simulink model "GLOSA_cs"  (Simulink 7.6)
settings: Solver=ode5  SolverMode=SingleTasking  StartTime=0.0  StopTime=40  FixedStep=1/100  RelTol=1e-3
PreLoadFcn:
  | check_NUTS_defined;
  | 
  | assignin('base',['model_closed_' bdroot],false);
InitFcn:
  | PreScanExperimentInitFcn(bdroot)
StopFcn:
  | PreScanExperimentStopFcn(bdroot)
undefined symbols (not defined in the file or in its folder): acceleration, actorID, actorType, DiscIntNeverNeededParam, GPS_alt, GPS_lat, GPS_long, intmax, lightBarInUse, messageID, publicVehicleType, rotZ, sireneInUse, trafficLightPriority, turnAdvice, velocity, x_ini_VRML, y_ini_VRML, yawRate, z_ini_VRML
libraries: simulink x337, simulink_extras x42, PreScanSimulinkLibrary x38, PreScanUsefulBlocks x14, simulink_need_slupdate x6
(part 37 of 38)

== stateflow chart BMW_Z3_1/GLOSA/GLOSA ==
  state GLOSA [parallel]
    settings: executionOrder=1
    state dvVelocity
      (default) -> (junction 445) : /* Velocity */
      (junction 445) -> (junction 447) [order 1] : [ (vForward == 1)]
      (junction 447) -> (junction 457) : {dvVelocity = CAM_Velocity[i];}
          | /* If car is at the front desired velocity if cars are set to its value */
      (junction 445) -> (junction 391) [order 2] : {dvVelocity = desiredVelocity;}
      (junction 457) -> (junction 391)
      (junction 391) -> (junction 458)
    state AngleandDistance
      state vDistance [parallel]
        settings: executionOrder=2
        (junction 439) -> (junction 438) : {Distance = 6371000*c;}
            | /*Calculate distance to the object */
        (default) -> (junction 440) : {a = (sin((CAM_GPSLatitude[i]*d2r-selfGPSLatitude*d2r)/2 ))^2+ cos(selfGPSLatitude*d2r)*cos(CAM_GPSLatitude[i]*d2r)*(sin((CAM_GPSLongitude[i]*d2r-selfGPSLongitude*d2r)/2))^2;}
        (junction 440) -> (junction 439) : {c=2*atan2(sqrt(a), sqrt(1-a));}
      state vAngle [parallel]
        settings: executionOrder=1
        (default) -> (junction 441) : {Angle = (atan2(CAM_GPSLatitude[i]-selfGPSLatitude, CAM_GPSLongitude[i] - selfGPSLongitude))/d2r;}
            | /* Calculate angle to the object */
    state diVelocity
      (default) -> (junction 412) : /* Velocity */
      (junction 393) -> (junction 446)
      (junction 412) -> (junction 411) [order 1] : [ (iForward == 1)]
      (junction 412) -> (junction 393) [order 2] : {diVelocity = desiredVelocity;}
      (junction 411) -> (junction 444) : {diVelocity = (CAM_message[i] - 1000) /3.6;}
          | /* If sign is foreward, set desired infrastructure velocity to its limit */
      (junction 444) -> (junction 393)
    state vForward
      (junction 397) -> (junction 403) [order 1] : [((CAM_YawRate[i] > 2 || CAM_YawRate[i] <-2)  && (selfYawRate > 2 || selfYawRate < -2))]
      (junction 403) -> (junction 427) : {R1 = CAM_Velocity[i]/(CAM_YawRate[i]*d2r);
          | R2 = selfVelocity/(selfYawRate*d2r);
          | alfa = CAM_Heading[i]-selfHeading;
          | L = 2*d2r*180*R2*(alfa/360);}
      (junction 464) -> (junction 449)
      (junction 448) -> (junction 464) : {bend = 1;}
          | /* Cars are on the same bent if both have non-zero yaw rate and estimated agle at detected distance of the host car is similar to the angle of detected vehicle */
      (junction 427) -> (junction 449) [order 2] : {bend = 2;}
      (junction 387) -> (junction 390)
      (junction 401) -> (junction 388) [order 2]
      (junction 449) -> (junction 402)
      (junction 402) -> (junction 401)
      (junction 390) -> (junction 388) [order 2]
      (default) -> (junction 397)
      (junction 394) -> (junction 395) [order 1] : [abs((Angle-selfHeading)%%360)<45 || abs((Angle-selfHeading)%%360)>315 || bend == 1]
      (junction 443) -> (junction 435) : {vForward = 0;}
      (junction 395) -> (junction 396) : {vForward = 1;}
          | /* Car is at the front if it will be reached in 2s and angles of cars are similar or they are on the bend */
      (junction 401) -> (junction 387) [order 1] : [(abs(CAM_Heading[i]-selfHeading)<45 || abs(CAM_Heading[i]-selfHeading)>315) || bend == 1 ]
      (junction 394) -> (junction 443) [order 2]
      (junction 390) -> (junction 392) [order 1] : [Distance < 100 && (selfVelocity - CAM_Velocity[i]) > 0 &&((Distance / (selfVelocity - CAM_Velocity[i])) < 2) && (abs(selfGPSAltitude - CAM_GPSAltitude[i]) < 10)]
      (junction 397) -> (junction 402) [order 2] : {bend = 0;}
      (junction 392) -> (junction 394)
      (junction 388) -> (junction 443)
      (junction 427) -> (junction 448) [order 1] : [((abs(L/selfVelocity*selfYawRate + selfHeading - CAM_Heading[i]) < 45) || (abs(L/selfVelocity*selfYawRate + selfHeading - CAM_Heading[i]) > 315)) ]
    state Velocity
      (junction 417) -> (junction 415) [order 2]
      (junction 417) -> (junction 416) [order 1] : [(dvVelocity<diVelocity) && (dvVelocity < desiredVelocity) && (vForward == 1)]
      (junction 419) -> (junction 417)
      (junction 418) -> (junction 419)
      (junction 433) -> (junction 418) : {Velocity = diVelocity;}
          | /* When Velocity from the sign is smaller then actual velocity of the car, Desired host velocity is set to the sign limit */
      (junction 434) -> (junction 419) [order 2] : {Velocity = desiredVelocity;}
      (junction 434) -> (junction 433) [order 1] : [(diVelocity<desiredVelocity)]
      (default) -> (junction 434) : /* Velocity */
      (junction 416) -> (junction 414) : {Velocity = dvVelocity;}
          | /* When detected car hes smaller velocity then host car and sign limits, desired velocity of host car is set to velocity of detected car */
      (junction 415) -> (junction 413) : {Velocity = min(dvVelocity, diVelocity, dlVelocity, desiredVelocity);}
      (junction 414) -> (junction 415)
    state lForward
      note: IF condition1 THEN action1
          | ELSE action2
          | ENDIF
      (junction 454) -> (junction 399) : {lForward = 0;}
      (junction 470) -> (junction 400) : {lForward = 1;}
          | /* Light recognized as foreward one, when it is less then 500m away and Angle to it less then 45 deg.*/
      (junction 452) -> (junction 470) [order 1] : [abs((Angle-selfHeading) %% 360)<45 || abs((Angle-selfHeading)%% 360)>315]
      (junction 452) -> (junction 454) [order 2]
      (junction 467) -> (junction 450) [order 2]
      (junction 451) -> (junction 450) [order 2]
      (junction 456) -> (junction 452)
      (junction 466) -> (junction 451)
      (junction 451) -> (junction 456) [order 1] : [Distance < 500 && (abs(selfGPSAltitude - CAM_GPSAltitude[i]) < 10)]
      (junction 450) -> (junction 454)
      (junction 467) -> (junction 466) [order 1] : [abs(CAM_Heading[i]-selfHeading)<45 || abs(CAM_Heading[i]-selfHeading)>315]
      (default) -> (junction 467)
    state iForward
      note: IF condition1 THEN action1
          | ELSE action2
          | ENDIF
      (junction 389) -> (junction 436) [order 1] : [Distance < 20 && (abs(selfGPSAltitude - CAM_GPSAltitude[i]) < 10)]
      (junction 398) -> (junction 424) [order 1] : [abs((Angle-selfHeading)%%360)<45 || abs((Angle-selfHeading)%%360)>315]
      (junction 389) -> (junction 423) [order 2]
      (junction 424) -> (junction 425) : {iForward = 1;}
          | /* Sign recognized as foreward one, when it is less then 20m away and Angle to it less then 45 deg.*/
      (junction 423) -> (junction 437)
      (junction 436) -> (junction 398)
      (junction 437) -> (junction 426) : {iForward = 0;}
      (junction 398) -> (junction 437) [order 2]
      (junction 442) -> (junction 432) [order 1] : [abs(CAM_Heading[i]-selfHeading)<45 || abs(CAM_Heading[i]-selfHeading)>315]
      (default) -> (junction 442)
      (junction 432) -> (junction 389)
      (junction 442) -> (junction 423) [order 2]
    state dlVelocity
      (junction 430) -> (junction 428) : {dlVelocity = max((Distance-7)/(CAM_warning[i]+20), 0);}
          | /*dlVelocity = Distance/CAM_warning[i];}*/
      (junction 461) -> (junction 431) [order 2]
      (junction 465) -> (junction 468)
      (junction 469) -> (junction 465)
      (junction 459) -> (junction 465) [order 2] : {dlVelocity=desiredVelocity;}
      (junction 455) -> (junction 453)
      (junction 463) -> (junction 461) [order 2]
      (junction 428) -> (junction 469)
      (junction 431) -> (junction 430) [order 2]
      (junction 431) -> (junction 429) [order 1] : [TTL<CAM_warning[i]]
      (junction 463) -> (junction 462) [order 1] : [CAM_trafficLightPriority[i] == 3]
      (junction 429) -> (junction 453) : {dlVelocity= desiredVelocity;}
      (junction 405) -> (junction 455)
      (junction 453) -> (junction 428)
      (junction 462) -> (junction 405) : {dlVelocity=desiredVelocity;}
      (junction 404) -> (junction 455) : {dlVelocity = max((Distance-2)/(CAM_warning[i]+0.5), 0);}
      (junction 461) -> (junction 404) [order 1] : [CAM_trafficLightPriority[i] == 1]
      (default) -> (junction 459) : /* Velocity */
      (junction 459) -> (junction 460) [order 1] : [ lForward == 1]
      (junction 460) -> (junction 463) : {TTL = Distance/selfVelocity;
          | Light = CAM_trafficLightPriority[i];
          | Time = CAM_warning[i];}
    lForward -> dlVelocity
    (junction 407) -> AngleandDistance
    (default) -> (junction 406) : {i =0;
        | vForward = 0;
        | iForward = 0;}
    (junction 406) -> (junction 407) [order 1] : [(vForward ==0 || iForward == 0) && i < CAM_Size]
        |  /*till sign and vehicle aren't detected*/
    (junction 408) -> iForward [order 2] : [iForward == 0 && (CAM_vehicleType[i] == 14 || CAM_vehicleType[i] == 15) && CAM_message[i] > 1000]
        | /* If no traffic sign are detected and Velocity of the sign isn't 0 */
    (junction 410) -> (junction 406) : {i = 0;
        | vForward = 0;
        | iForward = 0;
        | lForward = 0;}
    (junction 420) -> (junction 406) : {i++;}
    (junction 408) -> (junction 409) [order 3]
    (junction 406) -> Velocity [order 2]
    (junction 409) -> vForward [order 1] : [vForward == 0 && CAM_vehicleType[i] != 0]
        | /* If there was no nearer car at the front and Vehicle Type ID isn't 0 */
    (junction 409) -> (junction 420) [order 2]
    iForward -> diVelocity
    Velocity -> (junction 410)
    vForward -> dvVelocity
    dvVelocity -> (junction 422)
    diVelocity -> (junction 422)
    (junction 421) -> (junction 420)
    (junction 422) -> (junction 421)
    AngleandDistance -> (junction 408)
    (junction 408) -> lForward [order 1] : [lForward == 0 && CAM_trafficLightPriority[i] !=0]
    dlVelocity -> (junction 422)
  note: GLOSA Algorithms Based on CAM signal changed to continous
  data: R1 local double; R2 local double; a local double; alfa local double; bend local double; c local double; diVelocity local double =100; dvVelocity local double =100; i local double; iForward local double; L local double; vForward local double; dlVelocity local double =100; selfHeading input double; selfGPSLongitude input double; selfGPSLatitude input double; selfGPSAltitude input double; selfYawRate input double; selfVelocity input double; CAM_warning input double; CAM_message input double; CAM_vehicleType input double; Velocity output double; CAM_Velocity input double; CAM_YawRate input double; CAM_trafficLightPriority input double; CAM_GPSLatitude input double; CAM_GPSLongitude input double; CAM_GPSAltitude input double; CAM_Heading input double; CAM_Size input double; d2r constant double =pi()/180; desiredVelocity input double; TTL local double; lForward output double; Angle local double; Distance local double; Time output double; Light output double

== matlab function BMW_Z3_1/GLOSA/vehicle CAM generator/For Iterator Subsystem/Continuous signal ==
  data: CAM input double; CAM_o output double; CAM_old input double; i input double; NoMM input double; tmp_in input double; tmp_out output double
function [CAM_o, tmp_out] = con(CAM, CAM_old, i, NoMM, tmp_in)
%linearization and number of missed frames control
CAM_o = zeros(1, 16);
tmp_out = tmp_in(i);
if (CAM(i, 3) ~= 0)
    if (mod(tmp_in(i), NoMM+1) == 0)
        CAM_o = CAM (i, :);
    end
    tmp_out = tmp_in(i)+1;
else
    CAM_o = CAM_old(i, :);
end

== matlab function BMW_Z3_1/GLOSA/vehicle CAM generator/Seperate signals ==
  data: CAM_warning output double; CAM input double; CAM_messageID output double; CAM_vehicleID output double; CAM_vehicleType output double; CAM_publicVehicleType output double; CAM_lightBarInUse output double; CAM_sireneInUse output double; CAM_velocity output double; CAM_acceleration output double; CAM_yawRate output double; CAM_turnAdvice output double; CAM_trafficLightPriority output double; CAM_GPSlat output double; CAM_GPSlong output double; CAM_GPSalt output double; CAM_rotZ output double
function [CAM_warning, CAM_messageID, CAM_vehicleID, CAM_vehicleType, CAM_publicVehicleType, CAM_lightBarInUse, CAM_sireneInUse, CAM_velocity, CAM_acceleration, CAM_yawRate, CAM_turnAdvice, CAM_trafficLightPriority, CAM_GPSlat, CAM_GPSlong, CAM_GPSalt, CAM_rotZ] = fcn(CAM)
%Seperate signals

CAM_warning = CAM(:, 1);
CAM_messageID = CAM(:, 2); 
CAM_vehicleID = CAM(:, 3);
CAM_vehicleType = CAM(:, 4);
CAM_publicVehicleType = CAM(:, 5);
CAM_lightBarInUse = CAM(:, 6);
CAM_sireneInUse = CAM(:, 7);
CAM_velocity = CAM(:, 8);
CAM_acceleration = CAM(:, 9);
CAM_yawRate = CAM(:, 10);
CAM_turnAdvice = CAM(:, 11);
CAM_trafficLightPriority = CAM(:, 12);
CAM_GPSlat = CAM(:, 13);
CAM_GPSlong = CAM(:, 14);
CAM_GPSalt = CAM(:, 15);
CAM_rotZ = CAM(:, 16);

== matlab function BMW_Z3_1/GLOSA/vehicle CAM generator/Signal Matrix create ==
  data: CAM_warning input double; CAM output double; CAM_messageID input double; CAM_vehicleID input double; CAM_vehicleType input double; CAM_publicVehicleType input double; CAM_lightBarInUse input double; CAM_sireneInUse input double; CAM_velocity input double; CAM_acceleration input double; CAM_yawRate input double; CAM_turnAdvice input double; CAM_trafficLightPriority input double; CAM_GPSlat input double; CAM_GPSlong input double; CAM_GPSalt input double; CAM_rotZ input double
function CAM = fcn(CAM_warning, CAM_messageID, CAM_vehicleID, CAM_vehicleType, CAM_publicVehicleType, CAM_lightBarInUse, CAM_sireneInUse, CAM_velocity, CAM_acceleration, CAM_yawRate, CAM_turnAdvice, CAM_trafficLightPriority, CAM_GPSlat, CAM_GPSlong, CAM_GPSalt, CAM_rotZ)
%Signal Matrix creation

CAM = [CAM_warning, CAM_messageID, CAM_vehicleID, CAM_vehicleType, CAM_publicVehicleType, CAM_lightBarInUse, CAM_sireneInUse, CAM_velocity, CAM_acceleration, CAM_yawRate, CAM_turnAdvice, CAM_trafficLightPriority, CAM_GPSlat, CAM_GPSlong, CAM_GPSalt, CAM_rotZ];

== matlab function Toyota_Yaris_1/GLOSA/Embedded MATLAB Function ==
  data: u input double; y output double
function y = fcn(u)
%#eml

y = mod(u, 360);

== stateflow chart Toyota_Yaris_1/GLOSA/GLOSA ==
  state GLOSA [parallel]
    settings: executionOrder=1
    state dvVelocity
      (default) -> (junction 760) : /* Velocity */
      (junction 760) -> (junction 762) [order 1] : [ (vForward == 1)]
      (junction 762) -> (junction 772) : {dvVelocity = CAM_Velocity[i];}
          | /* If car is at the front desired velocity if cars are set to its value */
      (junction 760) -> (junction 706) [order 2] : {dvVelocity = desiredVelocity;}
      (junction 772) -> (junction 706)
      (junction 706) -> (junction 773)
    state AngleandDistance
      state vDistance [parallel]
        settings: executionOrder=2
        (junction 754) -> (junction 753) : {Distance = 6371000*c;}
            | /*Calculate distance to the object */
        (default) -> (junction 755) : {a = (sin((CAM_GPSLatitude[i]*d2r-selfGPSLatitude*d2r)/2 ))^2+ cos(selfGPSLatitude*d2r)*cos(CAM_GPSLatitude[i]*d2r)*(sin((CAM_GPSLongitude[i]*d2r-selfGPSLongitude*d2r)/2))^2;}
        (junction 755) -> (junction 754) : {c=2*atan2(sqrt(a), sqrt(1-a));}
      state vAngle [parallel]
        settings: executionOrder=1
        (default) -> (junction 756) : {Angle = (atan2(CAM_GPSLatitude[i]-selfGPSLatitude, CAM_GPSLongitude[i] - selfGPSLongitude))/d2r;}
            | /* Calculate angle to the object */
    state diVelocity
      (default) -> (junction 727) : /* Velocity */
      (junction 708) -> (junction 761)
      (junction 727) -> (junction 726) [order 1] : [ (iForward == 1)]
      (junction 727) -> (junction 708) [order 2] : {diVelocity = desiredVelocity;}
      (junction 726) -> (junction 759) : {diVelocity = (CAM_message[i] - 1000) /3.6;}
          | /* If sign is foreward, set desired infrastructure velocity to its limit */
      (junction 759) -> (junction 708)
    state vForward
      (junction 712) -> (junction 718) [order 1] : [((CAM_YawRate[i] > 2 || CAM_YawRate[i] <-2)  && (selfYawRate > 2 || selfYawRate < -2))]
      (junction 718) -> (junction 742) : {R1 = CAM_Velocity[i]/(CAM_YawRate[i]*d2r);
          | R2 = selfVelocity/(selfYawRate*d2r);
          | alfa = CAM_Heading[i]-selfHeading;
          | L = 2*d2r*180*R2*(alfa/360);}
      (junction 779) -> (junction 764)
      (junction 763) -> (junction 779) : {bend = 1;}
          | /* Cars are on the same bent if both have non-zero yaw rate and estimated agle at detected distance of the host car is similar to the angle of detected vehicle */
      (junction 742) -> (junction 764) [order 2] : {bend = 2;}
      (junction 702) -> (junction 705)
      (junction 716) -> (junction 703) [order 2]
      (junction 764) -> (junction 717)
      (junction 717) -> (junction 716)
      (junction 705) -> (junction 703) [order 2]
      (default) -> (junction 712)
      (junction 709) -> (junction 710) [order 1] : [abs((Angle-selfHeading)%%360)<45 || abs((Angle-selfHeading)%%360)>315 || bend == 1]
      (junction 758) -> (junction 750) : {vForward = 0;}
      (junction 710) -> (junction 711) : {vForward = 1;}
          | /* Car is at the front if it will be reached in 2s and angles of cars are similar or they are on the bend */
      (junction 716) -> (junction 702) [order 1] : [(abs(CAM_Heading[i]-selfHeading)<45 || abs(CAM_Heading[i]-selfHeading)>315) || bend == 1 ]
      (junction 709) -> (junction 758) [order 2]
      (junction 705) -> (junction 707) [order 1] : [Distance < 100 && (selfVelocity - CAM_Velocity[i]) > 0 &&((Distance / (selfVelocity - CAM_Velocity[i])) < 2) && (abs(selfGPSAltitude - CAM_GPSAltitude[i]) < 10)]
      (junction 712) -> (junction 717) [order 2] : {bend = 0;}
      (junction 707) -> (junction 709)
      (junction 703) -> (junction 758)
      (junction 742) -> (junction 763) [order 1] : [((abs(L/selfVelocity*selfYawRate + selfHeading - CAM_Heading[i]) < 45) || (abs(L/selfVelocity*selfYawRate + selfHeading - CAM_Heading[i]) > 315)) ]
    state Velocity
      (junction 732) -> (junction 730) [order 2]
      (junction 732) -> (junction 731) [order 1] : [(dvVelocity<diVelocity) && (dvVelocity < desiredVelocity) && (vForward == 1)]
      (junction 734) -> (junction 732)
      (junction 733) -> (junction 734)
      (junction 748) -> (junction 733) : {Velocity = diVelocity;}
          | /* When Velocity from the sign is smaller then actual velocity of the car, Desired host velocity is set to the sign limit */
      (junction 749) -> (junction 734) [order 2] : {Velocity = desiredVelocity;}
      (junction 749) -> (junction 748) [order 1] : [(diVelocity<desiredVelocity)]
      (default) -> (junction 749) : /* Velocity */
      (junction 731) -> (junction 729) : {Velocity = dvVelocity;}
          | /* When detected car hes smaller velocity then host car and sign limits, desired velocity of host car is set to velocity of detected car */
      (junction 730) -> (junction 728) : {Velocity = min(dvVelocity, diVelocity, dlVelocity, desiredVelocity);}
      (junction 729) -> (junction 730)
    state lForward
      note: IF condition1 THEN action1
          | ELSE action2
          | ENDIF
      (junction 769) -> (junction 714) : {lForward = 0;}
      (junction 785) -> (junction 715) : {lForward = 1;}
          | /* Light recognized as foreward one, when it is less then 500m away and Angle to it less then 45 deg.*/
      (junction 767) -> (junction 785) [order 1] : [abs((Angle-selfHeading) %% 360)<45 || abs((Angle-selfHeading)%% 360)>315]
      (junction 767) -> (junction 769) [order 2]
      (junction 782) -> (junction 765) [order 2]
      (junction 766) -> (junction 765) [order 2]
      (junction 771) -> (junction 767)
      (junction 781) -> (junction 766)
      (junction 766) -> (junction 771) [order 1] : [Distance < 500 && (abs(selfGPSAltitude - CAM_GPSAltitude[i]) < 10)]
      (junction 765) -> (junction 769)
      (junction 782) -> (junction 781) [order 1] : [abs(CAM_Heading[i]-selfHeading)<45 || abs(CAM_Heading[i]-selfHeading)>315]
      (default) -> (junction 782)
    state iForward
      note: IF condition1 THEN action1
          | ELSE action2
          | ENDIF
      (junction 704) -> (junction 751) [order 1] : [Distance < 20 && (abs(selfGPSAltitude - CAM_GPSAltitude[i]) < 10)]
      (junction 713) -> (junction 739) [order 1] : [abs((Angle-selfHeading)%%360)<45 || abs((Angle-selfHeading)%%360)>315]
      (junction 704) -> (junction 738) [order 2]
      (junction 739) -> (junction 740) : {iForward = 1;}
          | /* Sign recognized as foreward one, when it is less then 20m away and Angle to it less then 45 deg.*/
      (junction 738) -> (junction 752)
      (junction 751) -> (junction 713)
      (junction 752) -> (junction 741) : {iForward = 0;}
      (junction 713) -> (junction 752) [order 2]
      (junction 757) -> (junction 747) [order 1] : [abs(CAM_Heading[i]-selfHeading)<45 || abs(CAM_Heading[i]-selfHeading)>315]
      (default) -> (junction 757)
      (junction 747) -> (junction 704)
      (junction 757) -> (junction 738) [order 2]
    state dlVelocity
      (junction 745) -> (junction 743) : {dlVelocity = max((Distance-7)/(CAM_warning[i]+20), 0);}
          | /*dlVelocity = Distance/CAM_warning[i];}*/
      (junction 776) -> (junction 746) [order 2]
      (junction 780) -> (junction 783)
      (junction 784) -> (junction 780)
      (junction 774) -> (junction 780) [order 2] : {dlVelocity=desiredVelocity;}
      (junction 770) -> (junction 768)
      (junction 778) -> (junction 776) [order 2]
      (junction 743) -> (junction 784)
      (junction 746) -> (junction 745) [order 2]
      (junction 746) -> (junction 744) [order 1] : [TTL<CAM_warning[i]]
      (junction 778) -> (junction 777) [order 1] : [CAM_trafficLightPriority[i] == 3]
      (junction 744) -> (junction 768) : {dlVelocity= desiredVelocity;}
      (junction 720) -> (junction 770)
      (junction 768) -> (junction 743)
      (junction 777) -> (junction 720) : {dlVelocity=desiredVelocity;}
      (junction 719) -> (junction 770) : {dlVelocity = max((Distance-2)/(CAM_warning[i]+0.5), 0);}
      (junction 776) -> (junction 719) [order 1] : [CAM_trafficLightPriority[i] == 1]
      (default) -> (junction 774) : /* Velocity */
      (junction 774) -> (junction 775) [order 1] : [ lForward == 1]
      (junction 775) -> (junction 778) : {TTL = Distance/selfVelocity;
          | Light = CAM_trafficLightPriority[i];
          | Time = CAM_warning[i];}
    lForward -> dlVelocity
    (junction 722) -> AngleandDistance
    (default) -> (junction 721) : {i =0;
        | vForward = 0;
        | iForward = 0;}
    (junction 721) -> (junction 722) [order 1] : [(vForward ==0 || iForward == 0) && i < CAM_Size]
        |  /*till sign and vehicle aren't detected*/
    (junction 723) -> iForward [order 2] : [iForward == 0 && (CAM_vehicleType[i] == 14 || CAM_vehicleType[i] == 15) && CAM_message[i] > 1000]
        | /* If no traffic sign are detected and Velocity of the sign isn't 0 */
    (junction 725) -> (junction 721) : {i = 0;
        | vForward = 0;
        | iForward = 0;
        | lForward = 0;}
    (junction 735) -> (junction 721) : {i++;}
    (junction 723) -> (junction 724) [order 3]
    (junction 721) -> Velocity [order 2]
    (junction 724) -> vForward [order 1] : [vForward == 0 && CAM_vehicleType[i] != 0]
        | /* If there was no nearer car at the front and Vehicle Type ID isn't 0 */
    (junction 724) -> (junction 735) [order 2]
    iForward -> diVelocity
    Velocity -> (junction 725)
    vForward -> dvVelocity
    dvVelocity -> (junction 737)
    diVelocity -> (junction 737)
    (junction 736) -> (junction 735)
    (junction 737) -> (junction 736)
    AngleandDistance -> (junction 723)
    (junction 723) -> lForward [order 1] : [lForward == 0 && CAM_trafficLightPriority[i] !=0]
    dlVelocity -> (junction 737)
  note: GLOSA Algorithms Based on CAM signal changed to continous
  data: R1 local double; R2 local double; a local double; alfa local double; bend local double; c local double; diVelocity local double =100; dvVelocity local double =100; i local double; iForward local double; L local double; vForward local double; dlVelocity local double =100; selfHeading input double; selfGPSLongitude input double; selfGPSLatitude input double; selfGPSAltitude input double; selfYawRate input double; selfVelocity input double; CAM_warning input double; CAM_message input double; CAM_vehicleType input double; Velocity output double; CAM_Velocity input double; CAM_YawRate input double; CAM_trafficLightPriority input double; CAM_GPSLatitude input double; CAM_GPSLongitude input double; CAM_GPSAltitude input double; CAM_Heading input double; CAM_Size input double; d2r constant double =pi()/180; desiredVelocity input double; TTL local double; lForward output double; Angle local double; Distance local double; Time output double; Light output double

== matlab function Toyota_Yaris_1/GLOSA/vehicle CAM generator/For Iterator Subsystem/Continuous signal ==
  data: CAM input double; CAM_o output double; CAM_old input double; i input double; NoMM input double; tmp_in input double; tmp_out output double
function [CAM_o, tmp_out] = con(CAM, CAM_old, i, NoMM, tmp_in)
%linearization and number of missed frames control
CAM_o = zeros(1, 16);
tmp_out = tmp_in(i);
if (CAM(i, 3) ~= 0)
    if (mod(tmp_in(i), NoMM+1) == 0)
        CAM_o = CAM (i, :);
    end
    tmp_out = tmp_in(i)+1;
else
    CAM_o = CAM_old(i, :);
end

== matlab function Toyota_Yaris_1/GLOSA/vehicle CAM generator/Seperate signals ==
  data: CAM_warning output double; CAM input double; CAM_messageID output double; CAM_vehicleID output double; CAM_vehicleType output double; CAM_publicVehicleType output double; CAM_lightBarInUse output double; CAM_sireneInUse output double; CAM_velocity output double; CAM_acceleration output double; CAM_yawRate output double; CAM_turnAdvice output double; CAM_trafficLightPriority output double; CAM_GPSlat output double; CAM_GPSlong output double; CAM_GPSalt output double; CAM_rotZ output double
function [CAM_warning, CAM_messageID, CAM_vehicleID, CAM_vehicleType, CAM_publicVehicleType, CAM_lightBarInUse, CAM_sireneInUse, CAM_velocity, CAM_acceleration, CAM_yawRate, CAM_turnAdvice, CAM_trafficLightPriority, CAM_GPSlat, CAM_GPSlong, CAM_GPSalt, CAM_rotZ] = fcn(CAM)
%Seperate signals

CAM_warning = CAM(:, 1);
CAM_messageID = CAM(:, 2); 
CAM_vehicleID = CAM(:, 3);
CAM_vehicleType = CAM(:, 4);
CAM_publicVehicleType = CAM(:, 5);
CAM_lightBarInUse = CAM(:, 6);
CAM_sireneInUse = CAM(:, 7);
CAM_velocity = CAM(:, 8);
CAM_acceleration = CAM(:, 9);
CAM_yawRate = CAM(:, 10);
CAM_turnAdvice = CAM(:, 11);
CAM_trafficLightPriority = CAM(:, 12);
CAM_GPSlat = CAM(:, 13);
CAM_GPSlong = CAM(:, 14);
CAM_GPSalt = CAM(:, 15);
CAM_rotZ = CAM(:, 16);

== matlab function Toyota_Yaris_1/GLOSA/vehicle CAM generator/Signal Matrix create ==
  data: CAM_warning input double; CAM output double; CAM_messageID input double; CAM_vehicleID input double; CAM_vehicleType input double; CAM_publicVehicleType input double; CAM_lightBarInUse input double; CAM_sireneInUse input double; CAM_velocity input double; CAM_acceleration input double; CAM_yawRate input double; CAM_turnAdvice input double; CAM_trafficLightPriority input double; CAM_GPSlat input double; CAM_GPSlong input double; CAM_GPSalt input double; CAM_rotZ input double
function CAM = fcn(CAM_warning, CAM_messageID, CAM_vehicleID, CAM_vehicleType, CAM_publicVehicleType, CAM_lightBarInUse, CAM_sireneInUse, CAM_velocity, CAM_acceleration, CAM_yawRate, CAM_turnAdvice, CAM_trafficLightPriority, CAM_GPSlat, CAM_GPSlong, CAM_GPSalt, CAM_rotZ)
%Signal Matrix creation

CAM = [CAM_warning, CAM_messageID, CAM_vehicleID, CAM_vehicleType, CAM_publicVehicleType, CAM_lightBarInUse, CAM_sireneInUse, CAM_velocity, CAM_acceleration, CAM_yawRate, CAM_turnAdvice, CAM_trafficLightPriority, CAM_GPSlat, CAM_GPSlong, CAM_GPSalt, CAM_rotZ];

== matlab function Toyota_Previa_1/GLOSA/Embedded MATLAB Function ==
  data: u input double; y output double
function y = fcn(u)
%#eml

y = mod(u, 360);

== matlab function Mazda_RX8_1/GLOSA/Embedded MATLAB Function ==
  data: u input double; y output double
function y = fcn(u)
%#eml

y = mod(u, 360);

== stateflow chart Mazda_RX8_1/GLOSA/GLOSA ==
  state GLOSA [parallel]
    settings: executionOrder=1
    state dvVelocity
      (default) -> (junction 1082) : /* Velocity */
      (junction 1082) -> (junction 1084) [order 1] : [ (vForward == 1)]
      (junction 1084) -> (junction 1094) : {dvVelocity = CAM_Velocity[i];}
          | /* If car is at the front desired velocity if cars are set to its value */
      (junction 1082) -> (junction 1028) [order 2] : {dvVelocity = desiredVelocity;}
      (junction 1094) -> (junction 1028)
      (junction 1028) -> (junction 1095)
    state AngleandDistance
      state vDistance [parallel]
        settings: executionOrder=2
        (junction 1076) -> (junction 1075) : {Distance = 6371000*c;}
            | /*Calculate distance to the object */
        (default) -> (junction 1077) : {a = (sin((CAM_GPSLatitude[i]*d2r-selfGPSLatitude*d2r)/2 ))^2+ cos(selfGPSLatitude*d2r)*cos(CAM_GPSLatitude[i]*d2r)*(sin((CAM_GPSLongitude[i]*d2r-selfGPSLongitude*d2r)/2))^2;}
        (junction 1077) -> (junction 1076) : {c=2*atan2(sqrt(a), sqrt(1-a));}
      state vAngle [parallel]
        settings: executionOrder=1
        (default) -> (junction 1078) : {Angle = (atan2(CAM_GPSLatitude[i]-selfGPSLatitude, CAM_GPSLongitude[i] - selfGPSLongitude))/d2r;}
            | /* Calculate angle to the object */
    state diVelocity
      (default) -> (junction 1049) : /* Velocity */
      (junction 1030) -> (junction 1083)
      (junction 1049) -> (junction 1048) [order 1] : [ (iForward == 1)]
      (junction 1049) -> (junction 1030) [order 2] : {diVelocity = desiredVelocity;}
      (junction 1048) -> (junction 1081) : {diVelocity = (CAM_message[i] - 1000) /3.6;}
          | /* If sign is foreward, set desired infrastructure velocity to its limit */
      (junction 1081) -> (junction 1030)
    state vForward
      (junction 1034) -> (junction 1040) [order 1] : [((CAM_YawRate[i] > 2 || CAM_YawRate[i] <-2)  && (selfYawRate > 2 || selfYawRate < -2))]
      (junction 1040) -> (junction 1064) : {R1 = CAM_Velocity[i]/(CAM_YawRate[i]*d2r);
          | R2 = selfVelocity/(selfYawRate*d2r);
          | alfa = CAM_Heading[i]-selfHeading;
          | L = 2*d2r*180*R2*(alfa/360);}
      (junction 1101) -> (junction 1086)
      (junction 1085) -> (junction 1101) : {bend = 1;}
          | /* Cars are on the same bent if both have non-zero yaw rate and estimated agle at detected distance of the host car is similar to the angle of detected vehicle */
      (junction 1064) -> (junction 1086) [order 2] : {bend = 2;}
      (junction 1024) -> (junction 1027)
      (junction 1038) -> (junction 1025) [order 2]
      (junction 1086) -> (junction 1039)
      (junction 1039) -> (junction 1038)
      (junction 1027) -> (junction 1025) [order 2]
      (default) -> (junction 1034)
      (junction 1031) -> (junction 1032) [order 1] : [abs((Angle-selfHeading)%%360)<45 || abs((Angle-selfHeading)%%360)>315 || bend == 1]
      (junction 1080) -> (junction 1072) : {vForward = 0;}
      (junction 1032) -> (junction 1033) : {vForward = 1;}
          | /* Car is at the front if it will be reached in 2s and angles of cars are similar or they are on the bend */
      (junction 1038) -> (junction 1024) [order 1] : [(abs(CAM_Heading[i]-selfHeading)<45 || abs(CAM_Heading[i]-selfHeading)>315) || bend == 1 ]
      (junction 1031) -> (junction 1080) [order 2]
      (junction 1027) -> (junction 1029) [order 1] : [Distance < 100 && (selfVelocity - CAM_Velocity[i]) > 0 &&((Distance / (selfVelocity - CAM_Velocity[i])) < 2) && (abs(selfGPSAltitude - CAM_GPSAltitude[i]) < 10)]
      (junction 1034) -> (junction 1039) [order 2] : {bend = 0;}
      (junction 1029) -> (junction 1031)
      (junction 1025) -> (junction 1080)
      (junction 1064) -> (junction 1085) [order 1] : [((abs(L/selfVelocity*selfYawRate + selfHeading - CAM_Heading[i]) < 45) || (abs(L/selfVelocity*selfYawRate + selfHeading - CAM_Heading[i]) > 315)) ]
    state Velocity
      (junction 1054) -> (junction 1052) [order 2]
      (junction 1054) -> (junction 1053) [order 1] : [(dvVelocity<diVelocity) && (dvVelocity < desiredVelocity) && (vForward == 1)]
      (junction 1056) -> (junction 1054)
      (junction 1055) -> (junction 1056)
      (junction 1070) -> (junction 1055) : {Velocity = diVelocity;}
          | /* When Velocity from the sign is smaller then actual velocity of the car, Desired host velocity is set to the sign limit */
      (junction 1071) -> (junction 1056) [order 2] : {Velocity = desiredVelocity;}
      (junction 1071) -> (junction 1070) [order 1] : [(diVelocity<desiredVelocity)]
      (default) -> (junction 1071) : /* Velocity */
      (junction 1053) -> (junction 1051) : {Velocity = dvVelocity;}
          | /* When detected car hes smaller velocity then host car and sign limits, desired velocity of host car is set to velocity of detected car */
      (junction 1052) -> (junction 1050) : {Velocity = min(dvVelocity, diVelocity, dlVelocity, desiredVelocity);}
      (junction 1051) -> (junction 1052)
    state lForward
      note: IF condition1 THEN action1
          | ELSE action2
          | ENDIF
      (junction 1091) -> (junction 1036) : {lForward = 0;}
      (junction 1107) -> (junction 1037) : {lForward = 1;}
          | /* Light recognized as foreward one, when it is less then 500m away and Angle to it less then 45 deg.*/
      (junction 1089) -> (junction 1107) [order 1] : [abs((Angle-selfHeading) %% 360)<45 || abs((Angle-selfHeading)%% 360)>315]
      (junction 1089) -> (junction 1091) [order 2]
      (junction 1104) -> (junction 1087) [order 2]
      (junction 1088) -> (junction 1087) [order 2]
      (junction 1093) -> (junction 1089)
      (junction 1103) -> (junction 1088)
      (junction 1088) -> (junction 1093) [order 1] : [Distance < 500 && (abs(selfGPSAltitude - CAM_GPSAltitude[i]) < 10)]
      (junction 1087) -> (junction 1091)
      (junction 1104) -> (junction 1103) [order 1] : [abs(CAM_Heading[i]-selfHeading)<45 || abs(CAM_Heading[i]-selfHeading)>315]
      (default) -> (junction 1104)
    state iForward
      note: IF condition1 THEN action1
          | ELSE action2
          | ENDIF
      (junction 1026) -> (junction 1073) [order 1] : [Distance < 20 && (abs(selfGPSAltitude - CAM_GPSAltitude[i]) < 10)]
      (junction 1035) -> (junction 1061) [order 1] : [abs((Angle-selfHeading)%%360)<45 || abs((Angle-selfHeading)%%360)>315]
      (junction 1026) -> (junction 1060) [order 2]
      (junction 1061) -> (junction 1062) : {iForward = 1;}
          | /* Sign recognized as foreward one, when it is less then 20m away and Angle to it less then 45 deg.*/
      (junction 1060) -> (junction 1074)
      (junction 1073) -> (junction 1035)
      (junction 1074) -> (junction 1063) : {iForward = 0;}
      (junction 1035) -> (junction 1074) [order 2]
      (junction 1079) -> (junction 1069) [order 1] : [abs(CAM_Heading[i]-selfHeading)<45 || abs(CAM_Heading[i]-selfHeading)>315]
      (default) -> (junction 1079)
      (junction 1069) -> (junction 1026)
      (junction 1079) -> (junction 1060) [order 2]
    state dlVelocity
      (junction 1067) -> (junction 1065) : {dlVelocity = max((Distance-7)/(CAM_warning[i]+20), 0);}
          | /*dlVelocity = Distance/CAM_warning[i];}*/
      (junction 1098) -> (junction 1068) [order 2]
      (junction 1102) -> (junction 1105)
      (junction 1106) -> (junction 1102)
      (junction 1096) -> (junction 1102) [order 2] : {dlVelocity=desiredVelocity;}
      (junction 1092) -> (junction 1090)
      (junction 1100) -> (junction 1098) [order 2]
      (junction 1065) -> (junction 1106)
      (junction 1068) -> (junction 1067) [order 2]
      (junction 1068) -> (junction 1066) [order 1] : [TTL<CAM_warning[i]]
      (junction 1100) -> (junction 1099) [order 1] : [CAM_trafficLightPriority[i] == 3]
      (junction 1066) -> (junction 1090) : {dlVelocity= desiredVelocity;}
      (junction 1042) -> (junction 1092)
      (junction 1090) -> (junction 1065)
      (junction 1099) -> (junction 1042) : {dlVelocity=desiredVelocity;}
      (junction 1041) -> (junction 1092) : {dlVelocity = max((Distance-2)/(CAM_warning[i]+0.5), 0);}
      (junction 1098) -> (junction 1041) [order 1] : [CAM_trafficLightPriority[i] == 1]
      (default) -> (junction 1096) : /* Velocity */
      (junction 1096) -> (junction 1097) [order 1] : [ lForward == 1]
      (junction 1097) -> (junction 1100) : {TTL = Distance/selfVelocity;
          | Light = CAM_trafficLightPriority[i];
          | Time = CAM_warning[i];}
    lForward -> dlVelocity
    (junction 1044) -> AngleandDistance
    (default) -> (junction 1043) : {i =0;
        | vForward = 0;
        | iForward = 0;}
    (junction 1043) -> (junction 1044) [order 1] : [(vForward ==0 || iForward == 0) && i < CAM_Size]
        |  /*till sign and vehicle aren't detected*/
    (junction 1045) -> iForward [order 2] : [iForward == 0 && (CAM_vehicleType[i] == 14 || CAM_vehicleType[i] == 15) && CAM_message[i] > 1000]
        | /* If no traffic sign are detected and Velocity of the sign isn't 0 */
    (junction 1047) -> (junction 1043) : {i = 0;
        | vForward = 0;
        | iForward = 0;
        | lForward = 0;}
    (junction 1057) -> (junction 1043) : {i++;}
    (junction 1045) -> (junction 1046) [order 3]
    (junction 1043) -> Velocity [order 2]
    (junction 1046) -> vForward [order 1] : [vForward == 0 && CAM_vehicleType[i] != 0]
        | /* If there was no nearer car at the front and Vehicle Type ID isn't 0 */
    (junction 1046) -> (junction 1057) [order 2]
    iForward -> diVelocity
    Velocity -> (junction 1047)
    vForward -> dvVelocity
    dvVelocity -> (junction 1059)
    diVelocity -> (junction 1059)
    (junction 1058) -> (junction 1057)
    (junction 1059) -> (junction 1058)
    AngleandDistance -> (junction 1045)
    (junction 1045) -> lForward [order 1] : [lForward == 0 && CAM_trafficLightPriority[i] !=0]
    dlVelocity -> (junction 1059)
  note: GLOSA Algorithms Based on CAM signal changed to continous
  data: R1 local double; R2 local double; a local double; alfa local double; bend local double; c local double; diVelocity local double =100; dvVelocity local double =100; i local double; iForward local double; L local double; vForward local double; dlVelocity local double =100; selfHeading input double; selfGPSLongitude input double; selfGPSLatitude input double; selfGPSAltitude input double; selfYawRate input double; selfVelocity input double; CAM_warning input double; CAM_message input double; CAM_vehicleType input double; Velocity output double; CAM_Velocity input double; CAM_YawRate input double; CAM_trafficLightPriority input double; CAM_GPSLatitude input double; CAM_GPSLongitude input double; CAM_GPSAltitude input double; CAM_Heading input double; CAM_Size input double; d2r constant double =pi()/180; desiredVelocity input double; TTL local double; lForward output double; Angle local double; Distance local double; Time output double; Light output double

== matlab function Mazda_RX8_1/GLOSA/vehicle CAM generator/For Iterator Subsystem/Continuous signal ==
  data: CAM input double; CAM_o output double; CAM_old input double; i input double; NoMM input double; tmp_in input double; tmp_out output double
function [CAM_o, tmp_out] = con(CAM, CAM_old, i, NoMM, tmp_in)
%linearization and number of missed frames control
CAM_o = zeros(1, 16);
tmp_out = tmp_in(i);
if (CAM(i, 3) ~= 0)
    if (mod(tmp_in(i), NoMM+1) == 0)
        CAM_o = CAM (i, :);
    end
    tmp_out = tmp_in(i)+1;
else
    CAM_o = CAM_old(i, :);
end

== matlab function Mazda_RX8_1/GLOSA/vehicle CAM generator/Seperate signals ==
  data: CAM_warning output double; CAM input double; CAM_messageID output double; CAM_vehicleID output double; CAM_vehicleType output double; CAM_publicVehicleType output double; CAM_lightBarInUse output double; CAM_sireneInUse output double; CAM_velocity output double; CAM_acceleration output double; CAM_yawRate output double; CAM_turnAdvice output double; CAM_trafficLightPriority output double; CAM_GPSlat output double; CAM_GPSlong output double; CAM_GPSalt output double; CAM_rotZ output double
function [CAM_warning, CAM_messageID, CAM_vehicleID, CAM_vehicleType, CAM_publicVehicleType, CAM_lightBarInUse, CAM_sireneInUse, CAM_velocity, CAM_acceleration, CAM_yawRate, CAM_turnAdvice, CAM_trafficLightPriority, CAM_GPSlat, CAM_GPSlong, CAM_GPSalt, CAM_rotZ] = fcn(CAM)
%Seperate signals

CAM_warning = CAM(:, 1);
CAM_messageID = CAM(:, 2); 
CAM_vehicleID = CAM(:, 3);
CAM_vehicleType = CAM(:, 4);
CAM_publicVehicleType = CAM(:, 5);
CAM_lightBarInUse = CAM(:, 6);
CAM_sireneInUse = CAM(:, 7);
CAM_velocity = CAM(:, 8);
CAM_acceleration = CAM(:, 9);
CAM_yawRate = CAM(:, 10);
CAM_turnAdvice = CAM(:, 11);
CAM_trafficLightPriority = CAM(:, 12);
CAM_GPSlat = CAM(:, 13);
CAM_GPSlong = CAM(:, 14);
CAM_GPSalt = CAM(:, 15);
CAM_rotZ = CAM(:, 16);
